simulink model "VirtualADAPT"  (Simulink 8.9)
settings: Solver=ode45  StartTime=0.0  StopTime=inf  RelTol=1e-3  EnableMultiTasking=on
PostLoadFcn:
  | FaultInjectionGUIHandle = FaultInjectionGUI;
InitFcn:
  | VirtualADAPTDataStructure
undefined symbols (not defined in the file or in its folder): ln
libraries: simulink x363
(part 9 of 26)

== system VirtualADAPT/VirtualADAPTv1/PowerDistribution/ACLoadBank1/RelayEY173/ParallelConn ==
  u(1) : Inport
  u(2) : Inport  Port=2
  u(3) : Inport  Port=3
  Adder : Sum  Inputs=-  SaturateOnIntegerOverflow=on  InputSameDT=on  OutDataTypeStr=Inherit: Same as first input
  Gain : Gain  Gain=-1  SaturateOnIntegerOverflow=on  OutDataTypeStr=Inherit: Same as input  ParamDataTypeStr=Inherit: Same as input
  Mux : Mux  Inputs=3
  OffGuard : Fcn  Expr=(!u(3))
  OnGuard : Fcn  Expr=(u(3))
  Product1 : Product  SaturateOnIntegerOverflow=on  RndMeth=Zero  InputSameDT=on  OutDataTypeStr=Inherit: Same as first input
  Product2 : Product  SaturateOnIntegerOverflow=on  RndMeth=Zero  InputSameDT=on  OutDataTypeStr=Inherit: Same as first input
  determineState : Subsystem
  y(1) : Outport
  y(2) : Outport  Port=2
  O : Outport  Port=3
  State : Outport  Port=4
  wires:
  Adder/1 -> Gain/1
  u(3)/1 -> Mux/3
  Mux/1 -> OnGuard/1, OffGuard/1
  OnGuard/1 -> determineState/1(In1)
  OffGuard/1 -> determineState/2(In2)
  u(1)/1 -> Product1/1
  determineState/1(Out1) -> Product1/2, Product2/2, State/1
  Product1/1 -> O/1, y(2)/1
  Gain/1 -> Product2/1
  Product2/1 -> y(1)/1
  u(2)/1 -> Adder/1

== system VirtualADAPT/VirtualADAPTv1/PowerDistribution/ACLoadBank1/RelayEY173/Relay ==
  u(1) : Inport
  u(2) : Inport  Port=2
  u(3) : Inport  Port=3
  Adder : Sum  Inputs=+  SaturateOnIntegerOverflow=on  InputSameDT=on  OutDataTypeStr=Inherit: Same as first input
  Gain : Gain  SaturateOnIntegerOverflow=on  OutDataTypeStr=Inherit: Same as input  ParamDataTypeStr=Inherit: Same as input
  Mux : Mux  Inputs=3
  OffGuard : Fcn  Expr=(!u(3))
  OnGuard : Fcn  Expr=(u(3))
  Product1 : Product  SaturateOnIntegerOverflow=on  RndMeth=Zero  InputSameDT=on  OutDataTypeStr=Inherit: Same as first input
  Product2 : Product  SaturateOnIntegerOverflow=on  RndMeth=Zero  InputSameDT=on  OutDataTypeStr=Inherit: Same as first input
  determineState : Subsystem
  y(1) : Outport
  y(2) : Outport  Port=2
  O : Outport  Port=3
  State : Outport  Port=4
  wires:
  Adder/1 -> Gain/1
  u(3)/1 -> Mux/3
  Mux/1 -> OnGuard/1, OffGuard/1
  OnGuard/1 -> determineState/1(In1)
  OffGuard/1 -> determineState/2(In2)
  u(1)/1 -> Product1/1
  determineState/1(Out1) -> Product1/2, Product2/2, State/1
  Product1/1 -> O/1, y(2)/1
  Gain/1 -> Product2/1
  Product2/1 -> y(1)/1
  u(2)/1 -> Adder/1

== system VirtualADAPT/VirtualADAPTv1/PowerDistribution/ACLoadBank1/RelayEY173/Sensor ==
  TrueValue : Inport
  MSe : Subsystem  mask{FP=Nominal, FT=0, FM=0}
      mask prompts: FP "Fault Profile:"; FT "Fault Injection Time:"; FM "Fault Magnitude/Slope/Bias/StuckAtValue"
      mask TypeOptions FP: Nominal | Abrupt | Incipient | Bias | StuckAt
      mask Display: image(imread('MSe.png','BackgroundColor',[1 1 1]))
  R : Subsystem  mask{R=1, FP=Nominal, FT=0, FM=0}
      mask prompts: R "Value of R:"; FP "Fault Profile:"; FT "Fault Injection Time:"; FM "Fault Magnitude/Slope"
      mask TypeOptions FP: Nominal | Abrupt | Incipient
      mask Display: image(imread('R.png','BackgroundColor',[1 1 1]))
  TrueValueMod : Subsystem
      mask Display: image(imread('fx.png','BackgroundColor',[1 1 1]))
  Value : Subsystem
      mask Display: image(imread('0.png','BackgroundColor',[1 1 1]))
  SensedValue : Outport
  wires:
  Value/1(y(1)) -> R/1(In)
  R/1(Out) -> Value/1(u(1))
  MSe/1(E) -> Value/2(u(2))
  Value/2(y(2)) -> MSe/1(F)
  TrueValueMod/1(y(1)) -> MSe/2(Se)
  TrueValue/1 -> TrueValueMod/1(u(1))
  Value/3(O) -> SensedValue/1

== system VirtualADAPT/VirtualADAPTv1/PowerDistribution/ACLoadBank1/RelayEY174/ParallelConn ==
  u(1) : Inport
  u(2) : Inport  Port=2
  u(3) : Inport  Port=3
  Adder : Sum  Inputs=-  SaturateOnIntegerOverflow=on  InputSameDT=on  OutDataTypeStr=Inherit: Same as first input
  Gain : Gain  Gain=-1  SaturateOnIntegerOverflow=on  OutDataTypeStr=Inherit: Same as input  ParamDataTypeStr=Inherit: Same as input
  Mux : Mux  Inputs=3
  OffGuard : Fcn  Expr=(!u(3))
  OnGuard : Fcn  Expr=(u(3))
  Product1 : Product  SaturateOnIntegerOverflow=on  RndMeth=Zero  InputSameDT=on  OutDataTypeStr=Inherit: Same as first input
  Product2 : Product  SaturateOnIntegerOverflow=on  RndMeth=Zero  InputSameDT=on  OutDataTypeStr=Inherit: Same as first input
  determineState : Subsystem
  y(1) : Outport
  y(2) : Outport  Port=2
  O : Outport  Port=3
  State : Outport  Port=4
  wires:
  Adder/1 -> Gain/1
  u(3)/1 -> Mux/3
  Mux/1 -> OnGuard/1, OffGuard/1
  OnGuard/1 -> determineState/1(In1)
  OffGuard/1 -> determineState/2(In2)
  u(1)/1 -> Product1/1
  determineState/1(Out1) -> Product1/2, Product2/2, State/1
  Product1/1 -> O/1, y(2)/1
  Gain/1 -> Product2/1
  Product2/1 -> y(1)/1
  u(2)/1 -> Adder/1

== system VirtualADAPT/VirtualADAPTv1/PowerDistribution/ACLoadBank1/RelayEY174/Relay ==
  u(1) : Inport
  u(2) : Inport  Port=2
  u(3) : Inport  Port=3
  Adder : Sum  Inputs=+  SaturateOnIntegerOverflow=on  InputSameDT=on  OutDataTypeStr=Inherit: Same as first input
  Gain : Gain  SaturateOnIntegerOverflow=on  OutDataTypeStr=Inherit: Same as input  ParamDataTypeStr=Inherit: Same as input
  Mux : Mux  Inputs=3
  OffGuard : Fcn  Expr=(!u(3))
  OnGuard : Fcn  Expr=(u(3))
  Product1 : Product  SaturateOnIntegerOverflow=on  RndMeth=Zero  InputSameDT=on  OutDataTypeStr=Inherit: Same as first input
  Product2 : Product  SaturateOnIntegerOverflow=on  RndMeth=Zero  InputSameDT=on  OutDataTypeStr=Inherit: Same as first input
  determineState : Subsystem
  y(1) : Outport
  y(2) : Outport  Port=2
  O : Outport  Port=3
  State : Outport  Port=4
  wires:
  Adder/1 -> Gain/1
  u(3)/1 -> Mux/3
  Mux/1 -> OnGuard/1, OffGuard/1
  OnGuard/1 -> determineState/1(In1)
  OffGuard/1 -> determineState/2(In2)
  u(1)/1 -> Product1/1
  determineState/1(Out1) -> Product1/2, Product2/2, State/1
  Product1/1 -> O/1, y(2)/1
  Gain/1 -> Product2/1
  Product2/1 -> y(1)/1
  u(2)/1 -> Adder/1

== system VirtualADAPT/VirtualADAPTv1/PowerDistribution/ACLoadBank1/RelayEY174/Sensor ==
  TrueValue : Inport
  MSe : Subsystem  mask{FP=Nominal, FT=0, FM=0}
      mask prompts: FP "Fault Profile:"; FT "Fault Injection Time:"; FM "Fault Magnitude/Slope/Bias/StuckAtValue"
      mask TypeOptions FP: Nominal | Abrupt | Incipient | Bias | StuckAt
      mask Display: image(imread('MSe.png','BackgroundColor',[1 1 1]))
  R : Subsystem  mask{R=1, FP=Nominal, FT=0, FM=0}
      mask prompts: R "Value of R:"; FP "Fault Profile:"; FT "Fault Injection Time:"; FM "Fault Magnitude/Slope"
      mask TypeOptions FP: Nominal | Abrupt | Incipient
      mask Display: image(imread('R.png','BackgroundColor',[1 1 1]))
  TrueValueMod : Subsystem
      mask Display: image(imread('fx.png','BackgroundColor',[1 1 1]))
  Value : Subsystem
      mask Display: image(imread('0.png','BackgroundColor',[1 1 1]))
  SensedValue : Outport
  wires:
  Value/1(y(1)) -> R/1(In)
  R/1(Out) -> Value/1(u(1))
  MSe/1(E) -> Value/2(u(2))
  Value/2(y(2)) -> MSe/1(F)
  TrueValueMod/1(y(1)) -> MSe/2(Se)
  TrueValue/1 -> TrueValueMod/1(u(1))
  Value/3(O) -> SensedValue/1

== system VirtualADAPT/VirtualADAPTv1/PowerDistribution/ACLoadBank1/RelayEY175/ParallelConn ==
  u(1) : Inport
  u(2) : Inport  Port=2
  u(3) : Inport  Port=3
  Adder : Sum  Inputs=-  SaturateOnIntegerOverflow=on  InputSameDT=on  OutDataTypeStr=Inherit: Same as first input
  Gain : Gain  Gain=-1  SaturateOnIntegerOverflow=on  OutDataTypeStr=Inherit: Same as input  ParamDataTypeStr=Inherit: Same as input
  Mux : Mux  Inputs=3
  OffGuard : Fcn  Expr=(!u(3))
  OnGuard : Fcn  Expr=(u(3))
  Product1 : Product  SaturateOnIntegerOverflow=on  RndMeth=Zero  InputSameDT=on  OutDataTypeStr=Inherit: Same as first input
  Product2 : Product  SaturateOnIntegerOverflow=on  RndMeth=Zero  InputSameDT=on  OutDataTypeStr=Inherit: Same as first input
  determineState : Subsystem
  y(1) : Outport
  y(2) : Outport  Port=2
  O : Outport  Port=3
  State : Outport  Port=4
  wires:
  Adder/1 -> Gain/1
  u(3)/1 -> Mux/3
  Mux/1 -> OnGuard/1, OffGuard/1
  OnGuard/1 -> determineState/1(In1)
  OffGuard/1 -> determineState/2(In2)
  u(1)/1 -> Product1/1
  determineState/1(Out1) -> Product1/2, Product2/2, State/1
  Product1/1 -> O/1, y(2)/1
  Gain/1 -> Product2/1
  Product2/1 -> y(1)/1
  u(2)/1 -> Adder/1

== system VirtualADAPT/VirtualADAPTv1/PowerDistribution/ACLoadBank1/RelayEY175/Relay ==
  u(1) : Inport
  u(2) : Inport  Port=2
  u(3) : Inport  Port=3
  Adder : Sum  Inputs=+  SaturateOnIntegerOverflow=on  InputSameDT=on  OutDataTypeStr=Inherit: Same as first input
  Gain : Gain  SaturateOnIntegerOverflow=on  OutDataTypeStr=Inherit: Same as input  ParamDataTypeStr=Inherit: Same as input
  Mux : Mux  Inputs=3
  OffGuard : Fcn  Expr=(!u(3))
  OnGuard : Fcn  Expr=(u(3))
  Product1 : Product  SaturateOnIntegerOverflow=on  RndMeth=Zero  InputSameDT=on  OutDataTypeStr=Inherit: Same as first input
  Product2 : Product  SaturateOnIntegerOverflow=on  RndMeth=Zero  InputSameDT=on  OutDataTypeStr=Inherit: Same as first input
  determineState : Subsystem
  y(1) : Outport
  y(2) : Outport  Port=2
  O : Outport  Port=3
  State : Outport  Port=4
  wires:
  Adder/1 -> Gain/1
  u(3)/1 -> Mux/3
  Mux/1 -> OnGuard/1, OffGuard/1
  OnGuard/1 -> determineState/1(In1)
  OffGuard/1 -> determineState/2(In2)
  u(1)/1 -> Product1/1
  determineState/1(Out1) -> Product1/2, Product2/2, State/1
  Product1/1 -> O/1, y(2)/1
  Gain/1 -> Product2/1
  Product2/1 -> y(1)/1
  u(2)/1 -> Adder/1

== system VirtualADAPT/VirtualADAPTv1/PowerDistribution/ACLoadBank1/RelayEY175/Sensor ==
  TrueValue : Inport
  MSe : Subsystem  mask{FP=Nominal, FT=0, FM=0}
      mask prompts: FP "Fault Profile:"; FT "Fault Injection Time:"; FM "Fault Magnitude/Slope/Bias/StuckAtValue"
      mask TypeOptions FP: Nominal | Abrupt | Incipient | Bias | StuckAt
      mask Display: image(imread('MSe.png','BackgroundColor',[1 1 1]))
  R : Subsystem  mask{R=1, FP=Nominal, FT=0, FM=0}
      mask prompts: R "Value of R:"; FP "Fault Profile:"; FT "Fault Injection Time:"; FM "Fault Magnitude/Slope"
      mask TypeOptions FP: Nominal | Abrupt | Incipient
      mask Display: image(imread('R.png','BackgroundColor',[1 1 1]))
  TrueValueMod : Subsystem
      mask Display: image(imread('fx.png','BackgroundColor',[1 1 1]))
  Value : Subsystem
      mask Display: image(imread('0.png','BackgroundColor',[1 1 1]))
  SensedValue : Outport
  wires:
  Value/1(y(1)) -> R/1(In)
  R/1(Out) -> Value/1(u(1))
  MSe/1(E) -> Value/2(u(2))
  Value/2(y(2)) -> MSe/1(F)
  TrueValueMod/1(y(1)) -> MSe/2(Se)
  TrueValue/1 -> TrueValueMod/1(u(1))
  Value/3(O) -> SensedValue/1

== system VirtualADAPT/VirtualADAPTv1/PowerDistribution/ACLoadBank2/L2A/PMP1 ==
  InputIn0 : Inport
  GY : Subsystem  mask{GY=0.2231, FP=Nominal, FT=0, FM=0}
      mask prompts: GY "Value of GY:"; FP "Fault Profile:"; FT "Fault Injection Time:"; FM "Fault Magnitude/Slope"
      mask TypeOptions FP: Nominal | Abrupt | Incipient
      mask Display: image(imread('GY.png','BackgroundColor',[1 1 1]))
  Inductance : Subsystem  mask{I=44.449, IC=0, FP=Nominal, FT=0, FM=0}
      mask prompts: I "Value of I:"; IC "Initial Condition:"; FP "Fault Profile:"; FT "Fault Injection Time:"; FM "Fault Magnitude/Slope"
      mask TypeOptions FP: Nominal | Abrupt | Incipient
      mask Display: image(imread('I.png','BackgroundColor',[1 1 1]))
  Inertia : Subsystem  mask{I=0.00070624, IC=0, FP=Nominal, FT=0, FM=0}
      mask prompts: I "Value of I:"; IC "Initial Condition:"; FP "Fault Profile:"; FT "Fault Injection Time:"; FM "Fault Magnitude/Slope"
      mask TypeOptions FP: Nominal | Abrupt | Incipient
      mask Display: image(imread('I.png','BackgroundColor',[1 1 1]))
  MotorCurrent : Subsystem
      mask Display: image(imread('1.png','BackgroundColor',[1 1 1]))
  PumpFlow : Subsystem
      mask Display: image(imread('1.png','BackgroundColor',[1 1 1]))
  PumpResistance : Subsystem  mask{R=0.001748, FP=Nominal, FT=0, FM=0}
      mask prompts: R "Value of R:"; FP "Fault Profile:"; FT "Fault Injection Time:"; FM "Fault Magnitude/Slope"
      mask TypeOptions FP: Nominal | Abrupt | Incipient
      mask Display: image(imread('R.png','BackgroundColor',[1 1 1]))
  Resistance : Subsystem  mask{R=227.896, FP=Nominal, FT=0, FM=0}
      mask prompts: R "Value of R:"; FP "Fault Profile:"; FT "Fault Injection Time:"; FM "Fault Magnitude/Slope"
      mask TypeOptions FP: Nominal | Abrupt | Incipient
      mask Display: image(imread('R.png','BackgroundColor',[1 1 1]))
  InputOut0 : Outport
  Current : Outport  Port=2
  Flow : Outport  Port=3
  wires:
  MotorCurrent/1(y(1)) -> Inductance/1(E)
  Inductance/1(F) -> MotorCurrent/1(u(1))
  MotorCurrent/2(y(2)) -> GY/1(I1)
  GY/1(O1) -> MotorCurrent/2(u(2))
  GY/2(O2) -> PumpFlow/1(u(1))
  PumpFlow/1(y(1)) -> GY/2(I2)
  PumpFlow/2(y(2)) -> PumpResistance/1(In)
  PumpResistance/1(Out) -> PumpFlow/2(u(2))
  MotorCurrent/3(y(3)) -> Resistance/1(In)
  Resistance/1(Out) -> MotorCurrent/3(u(3))
  PumpFlow/3(y(3)) -> Inertia/1(E)
  Inertia/1(F) -> PumpFlow/3(u(3))
  InputIn0/1 -> MotorCurrent/4(u(4))
  MotorCurrent/4(y(4)) -> InputOut0/1
  PumpFlow/4(O) -> Flow/1
  MotorCurrent/5(O) -> Current/1

== system VirtualADAPT/VirtualADAPTv1/PowerDistribution/ACLoadBank2/L2A/SensorFT520 ==
  TrueValue : Inport
  Bias : Subsystem  mask{Constant=-0.04818, FP=Nominal, FT=0, FM=0}
      mask prompts: Constant "Value of Constant:"; FP "Fault Profile:"; FT "Fault Injection Time:"; FM "Fault Magnitude/Slope"
      mask TypeOptions FP: Nominal | Abrupt | Incipient
      mask Display: image(imread('Constant.png','BackgroundColor',[1 1 1]))
  Gain : Subsystem  mask{Constant=3.085, FP=Nominal, FT=0, FM=0}
      mask prompts: Constant "Value of Constant:"; FP "Fault Profile:"; FT "Fault Injection Time:"; FM "Fault Magnitude/Slope"
      mask TypeOptions FP: Nominal | Abrupt | Incipient
      mask Display: image(imread('Constant.png','BackgroundColor',[1 1 1]))
  MSe : Subsystem  mask{FP=Nominal, FT=0, FM=0}
      mask prompts: FP "Fault Profile:"; FT "Fault Injection Time:"; FM "Fault Magnitude/Slope/Bias/StuckAtValue"
      mask TypeOptions FP: Nominal | Abrupt | Incipient | Bias | StuckAt
      mask Display: image(imread('MSe.png','BackgroundColor',[1 1 1]))
  Mux : Mux  Inputs=3
  R : Subsystem  mask{R=1, FP=Nominal, FT=0, FM=0}
      mask prompts: R "Value of R:"; FP "Fault Profile:"; FT "Fault Injection Time:"; FM "Fault Magnitude/Slope"
      mask TypeOptions FP: Nominal | Abrupt | Incipient
      mask Display: image(imread('R.png','BackgroundColor',[1 1 1]))
  TrueValueMod : Subsystem
      mask Display: image(imread('fx.png','BackgroundColor',[1 1 1]))
  Value : Subsystem
      mask Display: image(imread('0.png','BackgroundColor',[1 1 1]))
  SensedValue : Outport
  wires:
  MSe/1(E) -> Value/1(u(1))
  Value/1(y(1)) -> MSe/1(F)
  Value/2(y(2)) -> R/1(In)
  R/1(Out) -> Value/2(u(2))
  TrueValueMod/1(y(1)) -> MSe/2(Se)
  Value/3(O) -> SensedValue/1
  TrueValue/1 -> Mux/1
  Gain/1(C) -> Mux/2
  Bias/1(C) -> Mux/3
  Mux/1 -> TrueValueMod/1(u(1))

== system VirtualADAPT/VirtualADAPTv1/PowerDistribution/ACLoadBank2/L2B/LGT2 ==
  EPIn0 : Inport
  AmbientTemp : Inport  Port=2
  ComputePower : Subsystem
      mask Display: image(imread('fx.png','BackgroundColor',[1 1 1]))
  I : Subsystem
      mask Display: image(imread('1.png','BackgroundColor',[1 1 1]))
  R : Subsystem  mask{R=230.47, FP=Nominal, FT=0, FM=0}
      mask prompts: R "Value of R:"; FP "Fault Profile:"; FT "Fault Injection Time:"; FM "Fault Magnitude/Slope"
      mask TypeOptions FP: Nominal | Abrupt | Incipient
      mask Display: image(imread('R.png','BackgroundColor',[1 1 1]))
  TemperatureModel : Subsystem
  V : Subsystem
      mask Display: image(imread('0.png','BackgroundColor',[1 1 1]))
  EPOut0 : Outport
  Power : Outport  Port=2
  Current : Outport  Port=3
  Temperature : Outport  Port=4
  wires:
  V/1(y(1)) -> R/1(In)
  R/1(Out) -> V/1(u(1))
  I/1(y(1)) -> V/2(u(2))
  V/2(y(2)) -> I/1(u(1))
  EPIn0/1 -> I/2(u(2))
  I/2(y(2)) -> EPOut0/1
  V/3(O) -> ComputePower/1(u(1))
  I/3(O) -> ComputePower/2(u(2)), Current/1
  ComputePower/1(y(1)) -> Power/1, TemperatureModel/2(Power)
  TemperatureModel/1(Temp) -> Temperature/1
  AmbientTemp/1 -> TemperatureModel/1(AmbientTemp)

== system VirtualADAPT/VirtualADAPTv1/PowerDistribution/ACLoadBank2/L2B/SensorTE510 ==
  TrueValue : Inport
  MSe : Subsystem  mask{FP=Nominal, FT=0, FM=0}
      mask prompts: FP "Fault Profile:"; FT "Fault Injection Time:"; FM "Fault Magnitude/Slope/Bias/StuckAtValue"
      mask TypeOptions FP: Nominal | Abrupt | Incipient | Bias | StuckAt
      mask Display: image(imread('MSe.png','BackgroundColor',[1 1 1]))
  R : Subsystem  mask{R=1, FP=Nominal, FT=0, FM=0}
      mask prompts: R "Value of R:"; FP "Fault Profile:"; FT "Fault Injection Time:"; FM "Fault Magnitude/Slope"
      mask TypeOptions FP: Nominal | Abrupt | Incipient
      mask Display: image(imread('R.png','BackgroundColor',[1 1 1]))
  TempBias : Subsystem  mask{Constant=-0.25, FP=Nominal, FT=0, FM=0}
      mask prompts: Constant "Value of Constant:"; FP "Fault Profile:"; FT "Fault Injection Time:"; FM "Fault Magnitude/Slope"
      mask TypeOptions FP: Nominal | Abrupt | Incipient
      mask Display: image(imread('Constant.png','BackgroundColor',[1 1 1]))
  TrueValueMod : Subsystem
      mask Display: image(imread('fx.png','BackgroundColor',[1 1 1]))
  Value : Subsystem
      mask Display: image(imread('0.png','BackgroundColor',[1 1 1]))
  SensedValue : Outport
  wires:
  MSe/1(E) -> Value/1(u(1))
  Value/1(y(1)) -> MSe/1(F)
  Value/2(y(2)) -> R/1(In)
  R/1(Out) -> Value/2(u(2))
  TrueValueMod/1(y(1)) -> MSe/2(Se)
  TempBias/1(C) -> TrueValueMod/1(u(1))
  TrueValue/1 -> TrueValueMod/2(u(2))
  Value/3(O) -> SensedValue/1

== system VirtualADAPT/VirtualADAPTv1/PowerDistribution/ACLoadBank2/L2C/FAN4 ==
  EPIn0 : Inport
  OneJunction : Subsystem
      mask Display: image(imread('1.png','BackgroundColor',[1 1 1]))
  R : Subsystem  mask{R=622.72, FP=Nominal, FT=0, FM=0}
      mask prompts: R "Value of R:"; FP "Fault Profile:"; FT "Fault Injection Time:"; FM "Fault Magnitude/Slope"
      mask TypeOptions FP: Nominal | Abrupt | Incipient
      mask Display: image(imread('R.png','BackgroundColor',[1 1 1]))
  ZeroJunction : Subsystem
      mask Display: image(imread('0.png','BackgroundColor',[1 1 1]))
  EPOut0 : Outport
  Current : Outport  Port=2
  Voltage : Outport  Port=3
  wires:
  OneJunction/1(y(1)) -> ZeroJunction/1(u(1))
  ZeroJunction/1(y(1)) -> OneJunction/1(u(1))
  ZeroJunction/2(y(2)) -> R/1(In)
  R/1(Out) -> ZeroJunction/2(u(2))
  EPIn0/1 -> OneJunction/2(u(2))
  OneJunction/2(y(2)) -> EPOut0/1
  OneJunction/3(O) -> Current/1
  ZeroJunction/3(O) -> Voltage/1

== system VirtualADAPT/VirtualADAPTv1/PowerDistribution/ACLoadBank2/L2D/LGT9 ==
  EPIn0 : Inport
  CurrentJunc : Subsystem
      mask Display: image(imread('1.png','BackgroundColor',[1 1 1]))
  R : Subsystem  mask{R=238, FP=Nominal, FT=0, FM=0}
      mask prompts: R "Value of R:"; FP "Fault Profile:"; FT "Fault Injection Time:"; FM "Fault Magnitude/Slope"
      mask TypeOptions FP: Nominal | Abrupt | Incipient
      mask Display: image(imread('R.png','BackgroundColor',[1 1 1]))
  VoltageJunc : Subsystem
      mask Display: image(imread('0.png','BackgroundColor',[1 1 1]))
  EPOut0 : Outport
  Voltage : Outport  Port=2
  Current : Outport  Port=3
  wires:
  CurrentJunc/1(y(1)) -> VoltageJunc/1(u(1))
  VoltageJunc/1(y(1)) -> CurrentJunc/1(u(1))
  VoltageJunc/2(y(2)) -> R/1(In)
  R/1(Out) -> VoltageJunc/2(u(2))
  EPIn0/1 -> CurrentJunc/2(u(2))
  CurrentJunc/2(y(2)) -> EPOut0/1
  VoltageJunc/3(O) -> Voltage/1
  CurrentJunc/3(O) -> Current/1

== system VirtualADAPT/VirtualADAPTv1/PowerDistribution/ACLoadBank2/L2E/LGT7 ==
  VInIn0 : Inport
  AmbientTemp : Inport  Port=2
  ComputeCurrent : Subsystem
      mask Display: image(imread('fx.png','BackgroundColor',[1 1 1]))
  ComputePower : Subsystem
      mask Display: image(imread('fx.png','BackgroundColor',[1 1 1]))
  LightBulb1 : Subsystem
  LightBulb2 : Subsystem
  LightBulb3 : Subsystem
  O1 : Subsystem
      mask Display: image(imread('1.png','BackgroundColor',[1 1 1]))
  Z1 : Subsystem
      mask Display: image(imread('0.png','BackgroundColor',[1 1 1]))
  VInOut0 : Outport
  Current : Outport  Port=2
  Power : Outport  Port=3
  Temperature1 : Outport  Port=4
  Temperature2 : Outport  Port=5
  Temperature3 : Outport  Port=6
  wires:
  O1/1(y(1)) -> Z1/1(u(1))
  Z1/1(y(1)) -> O1/1(u(1))
  LightBulb3/1(EPOut0) -> Z1/2(u(2))
  Z1/2(y(2)) -> LightBulb3/1(EPIn0)
  LightBulb2/1(EPOut0) -> Z1/3(u(3))
  Z1/3(y(3)) -> LightBulb2/1(EPIn0)
  LightBulb1/1(EPOut0) -> Z1/4(u(4))
  Z1/4(y(4)) -> LightBulb1/1(EPIn0)
  VInIn0/1 -> O1/2(u(2))
  O1/2(y(2)) -> VInOut0/1
  LightBulb3/3(Current) -> ComputeCurrent/1(u(1))
  LightBulb2/3(Power) -> ComputePower/1(u(1))
  LightBulb3/2(Power) -> ComputePower/2(u(2))
  LightBulb2/4(Current) -> ComputeCurrent/2(u(2))
  LightBulb1/3(Power) -> ComputePower/3(u(3))
  LightBulb1/4(Current) -> ComputeCurrent/3(u(3))
  ComputeCurrent/1(y(1)) -> Current/1
  ComputePower/1(y(1)) -> Power/1
  AmbientTemp/1 -> LightBulb2/2(AmbientTemp), LightBulb3/2(AmbientTemp), LightBulb1/2(AmbientTemp)
  LightBulb3/4(Temperature) -> Temperature3/1
  LightBulb2/2(Temperature) -> Temperature2/1
  LightBulb1/2(Temperature) -> Temperature1/1

== system VirtualADAPT/VirtualADAPTv1/PowerDistribution/ACLoadBank2/L2E/SensorLT505 ==
  Power : Inport
  Bias : Subsystem  mask{Constant=16.561, FP=Nominal, FT=0, FM=0}
      mask prompts: Constant "Value of Constant:"; FP "Fault Profile:"; FT "Fault Injection Time:"; FM "Fault Magnitude/Slope"
      mask TypeOptions FP: Nominal | Abrupt | Incipient
      mask Display: image(imread('Constant.png','BackgroundColor',[1 1 1]))
  ComputeLuminosity : Subsystem
      mask Display: image(imread('fx.png','BackgroundColor',[1 1 1]))
  Gain : Subsystem  mask{Constant=0.5854, FP=Nominal, FT=0, FM=0}
      mask prompts: Constant "Value of Constant:"; FP "Fault Profile:"; FT "Fault Injection Time:"; FM "Fault Magnitude/Slope"
      mask TypeOptions FP: Nominal | Abrupt | Incipient
      mask Display: image(imread('Constant.png','BackgroundColor',[1 1 1]))
  MSe : Subsystem  mask{FP=Nominal, FT=0, FM=0}
      mask prompts: FP "Fault Profile:"; FT "Fault Injection Time:"; FM "Fault Magnitude/Slope/Bias/StuckAtValue"
      mask TypeOptions FP: Nominal | Abrupt | Incipient | Bias | StuckAt
      mask Display: image(imread('MSe.png','BackgroundColor',[1 1 1]))
  R : Subsystem  mask{R=100, FP=Nominal, FT=0, FM=0}
      mask prompts: R "Value of R:"; FP "Fault Profile:"; FT "Fault Injection Time:"; FM "Fault Magnitude/Slope"
      mask TypeOptions FP: Nominal | Abrupt | Incipient
      mask Display: image(imread('R.png','BackgroundColor',[1 1 1]))
  ZeroJunction : Subsystem
      mask Display: image(imread('0.png','BackgroundColor',[1 1 1]))
  Light : Outport
  wires:
  ZeroJunction/1(y(1)) -> R/1(In)
  R/1(Out) -> ZeroJunction/1(u(1))
  MSe/1(E) -> ZeroJunction/2(u(2))
  ZeroJunction/2(y(2)) -> MSe/1(F)
  ComputeLuminosity/1(y(1)) -> MSe/2(Se)
  Gain/1(C) -> ComputeLuminosity/1(u(1))
  Power/1 -> ComputeLuminosity/2(u(2))
  Bias/1(C) -> ComputeLuminosity/3(u(3))
  ZeroJunction/3(O) -> Light/1

== system VirtualADAPT/VirtualADAPTv1/PowerDistribution/ACLoadBank2/L2E/SensorTE505 ==
  TrueValue : Inport
  MSe : Subsystem  mask{FP=Nominal, FT=0, FM=0}
      mask prompts: FP "Fault Profile:"; FT "Fault Injection Time:"; FM "Fault Magnitude/Slope/Bias/StuckAtValue"
      mask TypeOptions FP: Nominal | Abrupt | Incipient | Bias | StuckAt
      mask Display: image(imread('MSe.png','BackgroundColor',[1 1 1]))
  R : Subsystem  mask{R=1, FP=Nominal, FT=0, FM=0}
      mask prompts: R "Value of R:"; FP "Fault Profile:"; FT "Fault Injection Time:"; FM "Fault Magnitude/Slope"
      mask TypeOptions FP: Nominal | Abrupt | Incipient
      mask Display: image(imread('R.png','BackgroundColor',[1 1 1]))
  TempBias : Subsystem  mask{Constant=-0.45, FP=Nominal, FT=0, FM=0}
      mask prompts: Constant "Value of Constant:"; FP "Fault Profile:"; FT "Fault Injection Time:"; FM "Fault Magnitude/Slope"
      mask TypeOptions FP: Nominal | Abrupt | Incipient
      mask Display: image(imread('Constant.png','BackgroundColor',[1 1 1]))
  TrueValueMod : Subsystem
      mask Display: image(imread('fx.png','BackgroundColor',[1 1 1]))
  Value : Subsystem
      mask Display: image(imread('0.png','BackgroundColor',[1 1 1]))
  SensedValue : Outport
  wires:
  MSe/1(E) -> Value/1(u(1))
  Value/1(y(1)) -> MSe/1(F)
  Value/2(y(2)) -> R/1(In)
  R/1(Out) -> Value/2(u(2))
  TrueValueMod/1(y(1)) -> MSe/2(Se)
  TempBias/1(C) -> TrueValueMod/1(u(1))
  TrueValue/1 -> TrueValueMod/2(u(2))
  Value/3(O) -> SensedValue/1

== system VirtualADAPT/VirtualADAPTv1/PowerDistribution/ACLoadBank2/L2E/SensorTE506 ==
  TrueValue : Inport
  MSe : Subsystem  mask{FP=Nominal, FT=0, FM=0}
      mask prompts: FP "Fault Profile:"; FT "Fault Injection Time:"; FM "Fault Magnitude/Slope/Bias/StuckAtValue"
      mask TypeOptions FP: Nominal | Abrupt | Incipient | Bias | StuckAt
      mask Display: image(imread('MSe.png','BackgroundColor',[1 1 1]))
  R : Subsystem  mask{R=1, FP=Nominal, FT=0, FM=0}
      mask prompts: R "Value of R:"; FP "Fault Profile:"; FT "Fault Injection Time:"; FM "Fault Magnitude/Slope"
      mask TypeOptions FP: Nominal | Abrupt | Incipient
      mask Display: image(imread('R.png','BackgroundColor',[1 1 1]))
  TempBias : Subsystem  mask{Constant=-1, FP=Nominal, FT=0, FM=0}
      mask prompts: Constant "Value of Constant:"; FP "Fault Profile:"; FT "Fault Injection Time:"; FM "Fault Magnitude/Slope"
      mask TypeOptions FP: Nominal | Abrupt | Incipient
      mask Display: image(imread('Constant.png','BackgroundColor',[1 1 1]))
  TrueValueMod : Subsystem
      mask Display: image(imread('fx.png','BackgroundColor',[1 1 1]))
  Value : Subsystem
      mask Display: image(imread('0.png','BackgroundColor',[1 1 1]))
  SensedValue : Outport
  wires:
  MSe/1(E) -> Value/1(u(1))
  Value/1(y(1)) -> MSe/1(F)
  Value/2(y(2)) -> R/1(In)
  R/1(Out) -> Value/2(u(2))
  TrueValueMod/1(y(1)) -> MSe/2(Se)
  TempBias/1(C) -> TrueValueMod/1(u(1))
  TrueValue/1 -> TrueValueMod/2(u(2))
  Value/3(O) -> SensedValue/1

== system VirtualADAPT/VirtualADAPTv1/PowerDistribution/ACLoadBank2/L2E/SensorTE507 ==
  TrueValue : Inport
  MSe : Subsystem  mask{FP=Nominal, FT=0, FM=0}
      mask prompts: FP "Fault Profile:"; FT "Fault Injection Time:"; FM "Fault Magnitude/Slope/Bias/StuckAtValue"
      mask TypeOptions FP: Nominal | Abrupt | Incipient | Bias | StuckAt
      mask Display: image(imread('MSe.png','BackgroundColor',[1 1 1]))
  R : Subsystem  mask{R=1, FP=Nominal, FT=0, FM=0}
      mask prompts: R "Value of R:"; FP "Fault Profile:"; FT "Fault Injection Time:"; FM "Fault Magnitude/Slope"
      mask TypeOptions FP: Nominal | Abrupt | Incipient
      mask Display: image(imread('R.png','BackgroundColor',[1 1 1]))
  TempBias : Subsystem  mask{Constant=-0.73, FP=Nominal, FT=0, FM=0}
      mask prompts: Constant "Value of Constant:"; FP "Fault Profile:"; FT "Fault Injection Time:"; FM "Fault Magnitude/Slope"
      mask TypeOptions FP: Nominal | Abrupt | Incipient
      mask Display: image(imread('Constant.png','BackgroundColor',[1 1 1]))
  TrueValueMod : Subsystem
      mask Display: image(imread('fx.png','BackgroundColor',[1 1 1]))
  Value : Subsystem
      mask Display: image(imread('0.png','BackgroundColor',[1 1 1]))
  SensedValue : Outport
  wires:
  MSe/1(E) -> Value/1(u(1))
  Value/1(y(1)) -> MSe/1(F)
  Value/2(y(2)) -> R/1(In)
  R/1(Out) -> Value/2(u(2))
  TrueValueMod/1(y(1)) -> MSe/2(Se)
  TempBias/1(C) -> TrueValueMod/1(u(1))
  TrueValue/1 -> TrueValueMod/2(u(2))
  Value/3(O) -> SensedValue/1

== system VirtualADAPT/VirtualADAPTv1/PowerDistribution/ACLoadBank2/L2F/FAN2 ==
  InputIn0 : Inport
  FanRotationalVelocity : Subsystem
      mask Display: image(imread('1.png','BackgroundColor',[1 1 1]))
  Friction : Subsystem  mask{R=0.00017604, FP=Nominal, FT=0, FM=0}
      mask prompts: R "Value of R:"; FP "Fault Profile:"; FT "Fault Injection Time:"; FM "Fault Magnitude/Slope"
      mask TypeOptions FP: Nominal | Abrupt | Incipient
      mask Display: image(imread('R.png','BackgroundColor',[1 1 1]))
  GY : Subsystem  mask{GY=0.03114, FP=Nominal, FT=0, FM=0}
      mask prompts: GY "Value of GY:"; FP "Fault Profile:"; FT "Fault Injection Time:"; FM "Fault Magnitude/Slope"
      mask TypeOptions FP: Nominal | Abrupt | Incipient
      mask Display: image(imread('GY.png','BackgroundColor',[1 1 1]))
  Inductance : Subsystem  mask{I=16.064, IC=0, FP=Nominal, FT=0, FM=0}
      mask prompts: I "Value of I:"; IC "Initial Condition:"; FP "Fault Profile:"; FT "Fault Injection Time:"; FM "Fault Magnitude/Slope"
      mask TypeOptions FP: Nominal | Abrupt | Incipient
      mask Display: image(imread('I.png','BackgroundColor',[1 1 1]))
  Inertia : Subsystem  mask{I=0.0004527, IC=0, FP=Nominal, FT=0, FM=0}
      mask prompts: I "Value of I:"; IC "Initial Condition:"; FP "Fault Profile:"; FT "Fault Injection Time:"; FM "Fault Magnitude/Slope"
      mask TypeOptions FP: Nominal | Abrupt | Incipient
      mask Display: image(imread('I.png','BackgroundColor',[1 1 1]))
  MotorCurrent : Subsystem
      mask Display: image(imread('1.png','BackgroundColor',[1 1 1]))
  Resistance : Subsystem  mask{R=150.625, FP=Nominal, FT=0, FM=0}
      mask prompts: R "Value of R:"; FP "Fault Profile:"; FT "Fault Injection Time:"; FM "Fault Magnitude/Slope"
      mask TypeOptions FP: Nominal | Abrupt | Incipient
      mask Display: image(imread('R.png','BackgroundColor',[1 1 1]))
  InputOut0 : Outport
  RotationalVelocity : Outport  Port=2
  Current : Outport  Port=3
  wires:
  FanRotationalVelocity/1(y(1)) -> Friction/1(In)
  Friction/1(Out) -> FanRotationalVelocity/1(u(1))
  GY/1(O1) -> FanRotationalVelocity/2(u(2))
  FanRotationalVelocity/2(y(2)) -> GY/1(I1)
  MotorCurrent/1(y(1)) -> Resistance/1(In)
  Resistance/1(Out) -> MotorCurrent/1(u(1))
  MotorCurrent/2(y(2)) -> GY/2(I2)
  GY/2(O2) -> MotorCurrent/2(u(2))
  FanRotationalVelocity/3(y(3)) -> Inertia/1(E)
  Inertia/1(F) -> FanRotationalVelocity/3(u(3))
  MotorCurrent/3(y(3)) -> Inductance/1(E)
  Inductance/1(F) -> MotorCurrent/3(u(3))
  InputIn0/1 -> MotorCurrent/4(u(4))
  MotorCurrent/4(y(4)) -> InputOut0/1
  FanRotationalVelocity/4(O) -> RotationalVelocity/1
  MotorCurrent/5(O) -> Current/1

== system VirtualADAPT/VirtualADAPTv1/PowerDistribution/ACLoadBank2/L2F/SensorST516 ==
  TrueValue : Inport
  Bias : Subsystem  mask{Constant=0.66027, FP=Nominal, FT=0, FM=0}
      mask prompts: Constant "Value of Constant:"; FP "Fault Profile:"; FT "Fault Injection Time:"; FM "Fault Magnitude/Slope"
      mask TypeOptions FP: Nominal | Abrupt | Incipient
      mask Display: image(imread('Constant.png','BackgroundColor',[1 1 1]))
  Gain : Subsystem  mask{Constant=4.0435, FP=Nominal, FT=0, FM=0}
      mask prompts: Constant "Value of Constant:"; FP "Fault Profile:"; FT "Fault Injection Time:"; FM "Fault Magnitude/Slope"
      mask TypeOptions FP: Nominal | Abrupt | Incipient
      mask Display: image(imread('Constant.png','BackgroundColor',[1 1 1]))
  MSe : Subsystem  mask{FP=Nominal, FT=0, FM=0}
      mask prompts: FP "Fault Profile:"; FT "Fault Injection Time:"; FM "Fault Magnitude/Slope/Bias/StuckAtValue"
      mask TypeOptions FP: Nominal | Abrupt | Incipient | Bias | StuckAt
      mask Display: image(imread('MSe.png','BackgroundColor',[1 1 1]))
  Mux : Mux  Inputs=3
  R : Subsystem  mask{R=1, FP=Nominal, FT=0, FM=0}
      mask prompts: R "Value of R:"; FP "Fault Profile:"; FT "Fault Injection Time:"; FM "Fault Magnitude/Slope"
      mask TypeOptions FP: Nominal | Abrupt | Incipient
      mask Display: image(imread('R.png','BackgroundColor',[1 1 1]))
  TrueValueMod : Subsystem
      mask Display: image(imread('fx.png','BackgroundColor',[1 1 1]))
  Value : Subsystem
      mask Display: image(imread('0.png','BackgroundColor',[1 1 1]))
  SensedValue : Outport
  wires:
  MSe/1(E) -> Value/1(u(1))
  Value/1(y(1)) -> MSe/1(F)
  Value/2(y(2)) -> R/1(In)
  R/1(Out) -> Value/2(u(2))
  TrueValueMod/1(y(1)) -> MSe/2(Se)
  Value/3(O) -> SensedValue/1
  TrueValue/1 -> Mux/1
  Gain/1(C) -> Mux/2
  Bias/1(C) -> Mux/3
  Mux/1 -> TrueValueMod/1(u(1))

== system VirtualADAPT/VirtualADAPTv1/PowerDistribution/ACLoadBank2/RelayEY270/ParallelConn ==
  u(1) : Inport
  u(2) : Inport  Port=2
  u(3) : Inport  Port=3
  Adder : Sum  Inputs=-  SaturateOnIntegerOverflow=on  InputSameDT=on  OutDataTypeStr=Inherit: Same as first input
  Gain : Gain  Gain=-1  SaturateOnIntegerOverflow=on  OutDataTypeStr=Inherit: Same as input  ParamDataTypeStr=Inherit: Same as input
  Mux : Mux  Inputs=3
  OffGuard : Fcn  Expr=(!u(3))
  OnGuard : Fcn  Expr=(u(3))
  Product1 : Product  SaturateOnIntegerOverflow=on  RndMeth=Zero  InputSameDT=on  OutDataTypeStr=Inherit: Same as first input
  Product2 : Product  SaturateOnIntegerOverflow=on  RndMeth=Zero  InputSameDT=on  OutDataTypeStr=Inherit: Same as first input
  determineState : Subsystem
  y(1) : Outport
  y(2) : Outport  Port=2
  O : Outport  Port=3
  State : Outport  Port=4
  wires:
  Adder/1 -> Gain/1
  u(3)/1 -> Mux/3
  Mux/1 -> OnGuard/1, OffGuard/1
  OnGuard/1 -> determineState/1(In1)
  OffGuard/1 -> determineState/2(In2)
  u(1)/1 -> Product1/1
  determineState/1(Out1) -> Product1/2, Product2/2, State/1
  Product1/1 -> O/1, y(2)/1
  Gain/1 -> Product2/1
  Product2/1 -> y(1)/1
  u(2)/1 -> Adder/1

== system VirtualADAPT/VirtualADAPTv1/PowerDistribution/ACLoadBank2/RelayEY270/Relay ==
  u(1) : Inport
  u(2) : Inport  Port=2
  u(3) : Inport  Port=3
  Adder : Sum  Inputs=+  SaturateOnIntegerOverflow=on  InputSameDT=on  OutDataTypeStr=Inherit: Same as first input
  Gain : Gain  SaturateOnIntegerOverflow=on  OutDataTypeStr=Inherit: Same as input  ParamDataTypeStr=Inherit: Same as input
  Mux : Mux  Inputs=3
  OffGuard : Fcn  Expr=(!u(3))
  OnGuard : Fcn  Expr=(u(3))
  Product1 : Product  SaturateOnIntegerOverflow=on  RndMeth=Zero  InputSameDT=on  OutDataTypeStr=Inherit: Same as first input
  Product2 : Product  SaturateOnIntegerOverflow=on  RndMeth=Zero  InputSameDT=on  OutDataTypeStr=Inherit: Same as first input
  determineState : Subsystem
  y(1) : Outport
  y(2) : Outport  Port=2
  O : Outport  Port=3
  State : Outport  Port=4
  wires:
  Adder/1 -> Gain/1
  u(3)/1 -> Mux/3
  Mux/1 -> OnGuard/1, OffGuard/1
  OnGuard/1 -> determineState/1(In1)
  OffGuard/1 -> determineState/2(In2)
  u(1)/1 -> Product1/1
  determineState/1(Out1) -> Product1/2, Product2/2, State/1
  Product1/1 -> O/1, y(2)/1
  Gain/1 -> Product2/1
  Product2/1 -> y(1)/1
  u(2)/1 -> Adder/1

== system VirtualADAPT/VirtualADAPTv1/PowerDistribution/ACLoadBank2/RelayEY270/Sensor ==
  TrueValue : Inport
  MSe : Subsystem  mask{FP=Nominal, FT=0, FM=0}
      mask prompts: FP "Fault Profile:"; FT "Fault Injection Time:"; FM "Fault Magnitude/Slope/Bias/StuckAtValue"
      mask TypeOptions FP: Nominal | Abrupt | Incipient | Bias | StuckAt
      mask Display: image(imread('MSe.png','BackgroundColor',[1 1 1]))
  R : Subsystem  mask{R=1, FP=Nominal, FT=0, FM=0}
      mask prompts: R "Value of R:"; FP "Fault Profile:"; FT "Fault Injection Time:"; FM "Fault Magnitude/Slope"
      mask TypeOptions FP: Nominal | Abrupt | Incipient
      mask Display: image(imread('R.png','BackgroundColor',[1 1 1]))
  TrueValueMod : Subsystem
      mask Display: image(imread('fx.png','BackgroundColor',[1 1 1]))
  Value : Subsystem
      mask Display: image(imread('0.png','BackgroundColor',[1 1 1]))
  SensedValue : Outport
  wires:
  Value/1(y(1)) -> R/1(In)
  R/1(Out) -> Value/1(u(1))
  MSe/1(E) -> Value/2(u(2))
  Value/2(y(2)) -> MSe/1(F)
  TrueValueMod/1(y(1)) -> MSe/2(Se)
  TrueValue/1 -> TrueValueMod/1(u(1))
  Value/3(O) -> SensedValue/1

== system VirtualADAPT/VirtualADAPTv1/PowerDistribution/ACLoadBank2/RelayEY271/ParallelConn ==
  u(1) : Inport
  u(2) : Inport  Port=2
  u(3) : Inport  Port=3
  Adder : Sum  Inputs=-  SaturateOnIntegerOverflow=on  InputSameDT=on  OutDataTypeStr=Inherit: Same as first input
  Gain : Gain  Gain=-1  SaturateOnIntegerOverflow=on  OutDataTypeStr=Inherit: Same as input  ParamDataTypeStr=Inherit: Same as input
  Mux : Mux  Inputs=3
  OffGuard : Fcn  Expr=(!u(3))
  OnGuard : Fcn  Expr=(u(3))
  Product1 : Product  SaturateOnIntegerOverflow=on  RndMeth=Zero  InputSameDT=on  OutDataTypeStr=Inherit: Same as first input
  Product2 : Product  SaturateOnIntegerOverflow=on  RndMeth=Zero  InputSameDT=on  OutDataTypeStr=Inherit: Same as first input
  determineState : Subsystem
  y(1) : Outport
  y(2) : Outport  Port=2
  O : Outport  Port=3
  State : Outport  Port=4
  wires:
  Adder/1 -> Gain/1
  u(3)/1 -> Mux/3
  Mux/1 -> OnGuard/1, OffGuard/1
  OnGuard/1 -> determineState/1(In1)
  OffGuard/1 -> determineState/2(In2)
  u(1)/1 -> Product1/1
  determineState/1(Out1) -> Product1/2, Product2/2, State/1
  Product1/1 -> O/1, y(2)/1
  Gain/1 -> Product2/1
  Product2/1 -> y(1)/1
  u(2)/1 -> Adder/1

== system VirtualADAPT/VirtualADAPTv1/PowerDistribution/ACLoadBank2/RelayEY271/Relay ==
  u(1) : Inport
  u(2) : Inport  Port=2
  u(3) : Inport  Port=3
  Adder : Sum  Inputs=+  SaturateOnIntegerOverflow=on  InputSameDT=on  OutDataTypeStr=Inherit: Same as first input
  Gain : Gain  SaturateOnIntegerOverflow=on  OutDataTypeStr=Inherit: Same as input  ParamDataTypeStr=Inherit: Same as input
  Mux : Mux  Inputs=3
  OffGuard : Fcn  Expr=(!u(3))
  OnGuard : Fcn  Expr=(u(3))
  Product1 : Product  SaturateOnIntegerOverflow=on  RndMeth=Zero  InputSameDT=on  OutDataTypeStr=Inherit: Same as first input
  Product2 : Product  SaturateOnIntegerOverflow=on  RndMeth=Zero  InputSameDT=on  OutDataTypeStr=Inherit: Same as first input
  determineState : Subsystem
  y(1) : Outport
  y(2) : Outport  Port=2
  O : Outport  Port=3
  State : Outport  Port=4
  wires:
  Adder/1 -> Gain/1
  u(3)/1 -> Mux/3
  Mux/1 -> OnGuard/1, OffGuard/1
  OnGuard/1 -> determineState/1(In1)
  OffGuard/1 -> determineState/2(In2)
  u(1)/1 -> Product1/1
  determineState/1(Out1) -> Product1/2, Product2/2, State/1
  Product1/1 -> O/1, y(2)/1
  Gain/1 -> Product2/1
  Product2/1 -> y(1)/1
  u(2)/1 -> Adder/1

== system VirtualADAPT/VirtualADAPTv1/PowerDistribution/ACLoadBank2/RelayEY271/Sensor ==
  TrueValue : Inport
  MSe : Subsystem  mask{FP=Nominal, FT=0, FM=0}
      mask prompts: FP "Fault Profile:"; FT "Fault Injection Time:"; FM "Fault Magnitude/Slope/Bias/StuckAtValue"
      mask TypeOptions FP: Nominal | Abrupt | Incipient | Bias | StuckAt
      mask Display: image(imread('MSe.png','BackgroundColor',[1 1 1]))
  R : Subsystem  mask{R=1, FP=Nominal, FT=0, FM=0}
      mask prompts: R "Value of R:"; FP "Fault Profile:"; FT "Fault Injection Time:"; FM "Fault Magnitude/Slope"
      mask TypeOptions FP: Nominal | Abrupt | Incipient
      mask Display: image(imread('R.png','BackgroundColor',[1 1 1]))
  TrueValueMod : Subsystem
      mask Display: image(imread('fx.png','BackgroundColor',[1 1 1]))
  Value : Subsystem
      mask Display: image(imread('0.png','BackgroundColor',[1 1 1]))
  SensedValue : Outport
  wires:
  Value/1(y(1)) -> R/1(In)
  R/1(Out) -> Value/1(u(1))
  MSe/1(E) -> Value/2(u(2))
  Value/2(y(2)) -> MSe/1(F)
  TrueValueMod/1(y(1)) -> MSe/2(Se)
  TrueValue/1 -> TrueValueMod/1(u(1))
  Value/3(O) -> SensedValue/1

== system VirtualADAPT/VirtualADAPTv1/PowerDistribution/ACLoadBank2/RelayEY272/ParallelConn ==
  u(1) : Inport
  u(2) : Inport  Port=2
  u(3) : Inport  Port=3
  Adder : Sum  Inputs=-  SaturateOnIntegerOverflow=on  InputSameDT=on  OutDataTypeStr=Inherit: Same as first input
  Gain : Gain  Gain=-1  SaturateOnIntegerOverflow=on  OutDataTypeStr=Inherit: Same as input  ParamDataTypeStr=Inherit: Same as input
  Mux : Mux  Inputs=3
  OffGuard : Fcn  Expr=(!u(3))
  OnGuard : Fcn  Expr=(u(3))
  Product1 : Product  SaturateOnIntegerOverflow=on  RndMeth=Zero  InputSameDT=on  OutDataTypeStr=Inherit: Same as first input
  Product2 : Product  SaturateOnIntegerOverflow=on  RndMeth=Zero  InputSameDT=on  OutDataTypeStr=Inherit: Same as first input
  determineState : Subsystem
  y(1) : Outport
  y(2) : Outport  Port=2
  O : Outport  Port=3
  State : Outport  Port=4
  wires:
  Adder/1 -> Gain/1
  u(3)/1 -> Mux/3
  Mux/1 -> OnGuard/1, OffGuard/1
  OnGuard/1 -> determineState/1(In1)
  OffGuard/1 -> determineState/2(In2)
  u(1)/1 -> Product1/1
  determineState/1(Out1) -> Product1/2, Product2/2, State/1
  Product1/1 -> O/1, y(2)/1
  Gain/1 -> Product2/1
  Product2/1 -> y(1)/1
  u(2)/1 -> Adder/1

== system VirtualADAPT/VirtualADAPTv1/PowerDistribution/ACLoadBank2/RelayEY272/Relay ==
  u(1) : Inport
  u(2) : Inport  Port=2
  u(3) : Inport  Port=3
  Adder : Sum  Inputs=+  SaturateOnIntegerOverflow=on  InputSameDT=on  OutDataTypeStr=Inherit: Same as first input
  Gain : Gain  SaturateOnIntegerOverflow=on  OutDataTypeStr=Inherit: Same as input  ParamDataTypeStr=Inherit: Same as input
  Mux : Mux  Inputs=3
  OffGuard : Fcn  Expr=(!u(3))
  OnGuard : Fcn  Expr=(u(3))
  Product1 : Product  SaturateOnIntegerOverflow=on  RndMeth=Zero  InputSameDT=on  OutDataTypeStr=Inherit: Same as first input
  Product2 : Product  SaturateOnIntegerOverflow=on  RndMeth=Zero  InputSameDT=on  OutDataTypeStr=Inherit: Same as first input
  determineState : Subsystem
  y(1) : Outport
  y(2) : Outport  Port=2
  O : Outport  Port=3
  State : Outport  Port=4
  wires:
  Adder/1 -> Gain/1
  u(3)/1 -> Mux/3
  Mux/1 -> OnGuard/1, OffGuard/1
  OnGuard/1 -> determineState/1(In1)
  OffGuard/1 -> determineState/2(In2)
  u(1)/1 -> Product1/1
  determineState/1(Out1) -> Product1/2, Product2/2, State/1
  Product1/1 -> O/1, y(2)/1
  Gain/1 -> Product2/1
  Product2/1 -> y(1)/1
  u(2)/1 -> Adder/1

== system VirtualADAPT/VirtualADAPTv1/PowerDistribution/ACLoadBank2/RelayEY272/Sensor ==
  TrueValue : Inport
  MSe : Subsystem  mask{FP=Nominal, FT=0, FM=0}
      mask prompts: FP "Fault Profile:"; FT "Fault Injection Time:"; FM "Fault Magnitude/Slope/Bias/StuckAtValue"
      mask TypeOptions FP: Nominal | Abrupt | Incipient | Bias | StuckAt
      mask Display: image(imread('MSe.png','BackgroundColor',[1 1 1]))
  R : Subsystem  mask{R=1, FP=Nominal, FT=0, FM=0}
      mask prompts: R "Value of R:"; FP "Fault Profile:"; FT "Fault Injection Time:"; FM "Fault Magnitude/Slope"
      mask TypeOptions FP: Nominal | Abrupt | Incipient
      mask Display: image(imread('R.png','BackgroundColor',[1 1 1]))
  TrueValueMod : Subsystem
      mask Display: image(imread('fx.png','BackgroundColor',[1 1 1]))
  Value : Subsystem
      mask Display: image(imread('0.png','BackgroundColor',[1 1 1]))
  SensedValue : Outport
  wires:
  Value/1(y(1)) -> R/1(In)
  R/1(Out) -> Value/1(u(1))
  MSe/1(E) -> Value/2(u(2))
  Value/2(y(2)) -> MSe/1(F)
  TrueValueMod/1(y(1)) -> MSe/2(Se)
  TrueValue/1 -> TrueValueMod/1(u(1))
  Value/3(O) -> SensedValue/1

== system VirtualADAPT/VirtualADAPTv1/PowerDistribution/ACLoadBank2/RelayEY273/ParallelConn ==
  u(1) : Inport
  u(2) : Inport  Port=2
  u(3) : Inport  Port=3
  Adder : Sum  Inputs=-  SaturateOnIntegerOverflow=on  InputSameDT=on  OutDataTypeStr=Inherit: Same as first input
  Gain : Gain  Gain=-1  SaturateOnIntegerOverflow=on  OutDataTypeStr=Inherit: Same as input  ParamDataTypeStr=Inherit: Same as input
  Mux : Mux  Inputs=3
  OffGuard : Fcn  Expr=(!u(3))
  OnGuard : Fcn  Expr=(u(3))
  Product1 : Product  SaturateOnIntegerOverflow=on  RndMeth=Zero  InputSameDT=on  OutDataTypeStr=Inherit: Same as first input
  Product2 : Product  SaturateOnIntegerOverflow=on  RndMeth=Zero  InputSameDT=on  OutDataTypeStr=Inherit: Same as first input
  determineState : Subsystem
  y(1) : Outport
  y(2) : Outport  Port=2
  O : Outport  Port=3
  State : Outport  Port=4
  wires:
  Adder/1 -> Gain/1
  u(3)/1 -> Mux/3
  Mux/1 -> OnGuard/1, OffGuard/1
  OnGuard/1 -> determineState/1(In1)
  OffGuard/1 -> determineState/2(In2)
  u(1)/1 -> Product1/1
  determineState/1(Out1) -> Product1/2, Product2/2, State/1
  Product1/1 -> O/1, y(2)/1
  Gain/1 -> Product2/1
  Product2/1 -> y(1)/1
  u(2)/1 -> Adder/1

== system VirtualADAPT/VirtualADAPTv1/PowerDistribution/ACLoadBank2/RelayEY273/Relay ==
  u(1) : Inport
  u(2) : Inport  Port=2
  u(3) : Inport  Port=3
  Adder : Sum  Inputs=+  SaturateOnIntegerOverflow=on  InputSameDT=on  OutDataTypeStr=Inherit: Same as first input
  Gain : Gain  SaturateOnIntegerOverflow=on  OutDataTypeStr=Inherit: Same as input  ParamDataTypeStr=Inherit: Same as input
  Mux : Mux  Inputs=3
  OffGuard : Fcn  Expr=(!u(3))
  OnGuard : Fcn  Expr=(u(3))
  Product1 : Product  SaturateOnIntegerOverflow=on  RndMeth=Zero  InputSameDT=on  OutDataTypeStr=Inherit: Same as first input
  Product2 : Product  SaturateOnIntegerOverflow=on  RndMeth=Zero  InputSameDT=on  OutDataTypeStr=Inherit: Same as first input
  determineState : Subsystem
  y(1) : Outport
  y(2) : Outport  Port=2
  O : Outport  Port=3
  State : Outport  Port=4
  wires:
  Adder/1 -> Gain/1
  u(3)/1 -> Mux/3
  Mux/1 -> OnGuard/1, OffGuard/1
  OnGuard/1 -> determineState/1(In1)
  OffGuard/1 -> determineState/2(In2)
  u(1)/1 -> Product1/1
  determineState/1(Out1) -> Product1/2, Product2/2, State/1
  Product1/1 -> O/1, y(2)/1
  Gain/1 -> Product2/1
  Product2/1 -> y(1)/1
  u(2)/1 -> Adder/1

== system VirtualADAPT/VirtualADAPTv1/PowerDistribution/ACLoadBank2/RelayEY273/Sensor ==
  TrueValue : Inport
  MSe : Subsystem  mask{FP=Nominal, FT=0, FM=0}
      mask prompts: FP "Fault Profile:"; FT "Fault Injection Time:"; FM "Fault Magnitude/Slope/Bias/StuckAtValue"
      mask TypeOptions FP: Nominal | Abrupt | Incipient | Bias | StuckAt
      mask Display: image(imread('MSe.png','BackgroundColor',[1 1 1]))
  R : Subsystem  mask{R=1, FP=Nominal, FT=0, FM=0}
      mask prompts: R "Value of R:"; FP "Fault Profile:"; FT "Fault Injection Time:"; FM "Fault Magnitude/Slope"
      mask TypeOptions FP: Nominal | Abrupt | Incipient
      mask Display: image(imread('R.png','BackgroundColor',[1 1 1]))
  TrueValueMod : Subsystem
      mask Display: image(imread('fx.png','BackgroundColor',[1 1 1]))
  Value : Subsystem
      mask Display: image(imread('0.png','BackgroundColor',[1 1 1]))
  SensedValue : Outport
  wires:
  Value/1(y(1)) -> R/1(In)
  R/1(Out) -> Value/1(u(1))
  MSe/1(E) -> Value/2(u(2))
  Value/2(y(2)) -> MSe/1(F)
  TrueValueMod/1(y(1)) -> MSe/2(Se)
  TrueValue/1 -> TrueValueMod/1(u(1))
  Value/3(O) -> SensedValue/1

== system VirtualADAPT/VirtualADAPTv1/PowerDistribution/ACLoadBank2/RelayEY274/ParallelConn ==
  u(1) : Inport
  u(2) : Inport  Port=2
  u(3) : Inport  Port=3
  Adder : Sum  Inputs=-  SaturateOnIntegerOverflow=on  InputSameDT=on  OutDataTypeStr=Inherit: Same as first input
  Gain : Gain  Gain=-1  SaturateOnIntegerOverflow=on  OutDataTypeStr=Inherit: Same as input  ParamDataTypeStr=Inherit: Same as input
  Mux : Mux  Inputs=3
  OffGuard : Fcn  Expr=(!u(3))
  OnGuard : Fcn  Expr=(u(3))
  Product1 : Product  SaturateOnIntegerOverflow=on  RndMeth=Zero  InputSameDT=on  OutDataTypeStr=Inherit: Same as first input
  Product2 : Product  SaturateOnIntegerOverflow=on  RndMeth=Zero  InputSameDT=on  OutDataTypeStr=Inherit: Same as first input
  determineState : Subsystem
  y(1) : Outport
  y(2) : Outport  Port=2
  O : Outport  Port=3
  State : Outport  Port=4
  wires:
  Adder/1 -> Gain/1
  u(3)/1 -> Mux/3
  Mux/1 -> OnGuard/1, OffGuard/1
  OnGuard/1 -> determineState/1(In1)
  OffGuard/1 -> determineState/2(In2)
  u(1)/1 -> Product1/1
  determineState/1(Out1) -> Product1/2, Product2/2, State/1
  Product1/1 -> O/1, y(2)/1
  Gain/1 -> Product2/1
  Product2/1 -> y(1)/1
  u(2)/1 -> Adder/1

== system VirtualADAPT/VirtualADAPTv1/PowerDistribution/ACLoadBank2/RelayEY274/Relay ==
  u(1) : Inport
  u(2) : Inport  Port=2
  u(3) : Inport  Port=3
  Adder : Sum  Inputs=+  SaturateOnIntegerOverflow=on  InputSameDT=on  OutDataTypeStr=Inherit: Same as first input
  Gain : Gain  SaturateOnIntegerOverflow=on  OutDataTypeStr=Inherit: Same as input  ParamDataTypeStr=Inherit: Same as input
  Mux : Mux  Inputs=3
  OffGuard : Fcn  Expr=(!u(3))
  OnGuard : Fcn  Expr=(u(3))
  Product1 : Product  SaturateOnIntegerOverflow=on  RndMeth=Zero  InputSameDT=on  OutDataTypeStr=Inherit: Same as first input
  Product2 : Product  SaturateOnIntegerOverflow=on  RndMeth=Zero  InputSameDT=on  OutDataTypeStr=Inherit: Same as first input
  determineState : Subsystem
  y(1) : Outport
  y(2) : Outport  Port=2
  O : Outport  Port=3
  State : Outport  Port=4
  wires:
  Adder/1 -> Gain/1
  u(3)/1 -> Mux/3
  Mux/1 -> OnGuard/1, OffGuard/1
  OnGuard/1 -> determineState/1(In1)
  OffGuard/1 -> determineState/2(In2)
  u(1)/1 -> Product1/1
  determineState/1(Out1) -> Product1/2, Product2/2, State/1
  Product1/1 -> O/1, y(2)/1
  Gain/1 -> Product2/1
  Product2/1 -> y(1)/1
  u(2)/1 -> Adder/1
